simulink model "CC_CarExample_template_inclass"  (R2022b)
settings: Solver=VariableStepAuto  StartTime=0.0  StopTime=400  RelTol=1e-3
model workspace (MAT data in the model file):
  Logfile_Data_Object : robotics.ros.BagSelection (value not decoded)
  Logfile_Path = '/Users/sprinkle/Library/CloudStorage/OneDrive-Vanderbilt/Presentations/DW_JS/TEACHING/Lecture Slides/2023_09_28_16_19_25_2T3MWRFVXLW056972microstrain_record.bag'
  Logfile_Playback_Duration = nan
  Logfile_Playback_Offset = 100
libraries: robotlib x3
settings Model: Creator=sprinkle  LastModifiedBy=sprinkle
settings SolverCC: AlgebraicLoopSolver=TrustRegion
settings DebuggingCC: UnconnectedInputMsg=warning  UnconnectedOutputMsg=warning  UnconnectedLineMsg=warning  SFMachineParentedDataDiag=warning
settings ModelReferenceCC: EnableRefExpFcnMdlSchedulingChecks=on

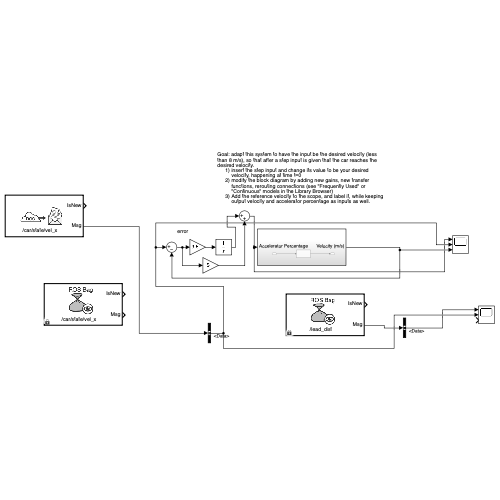
[thumbnail: Simulink's stored preview of the top-level diagram]
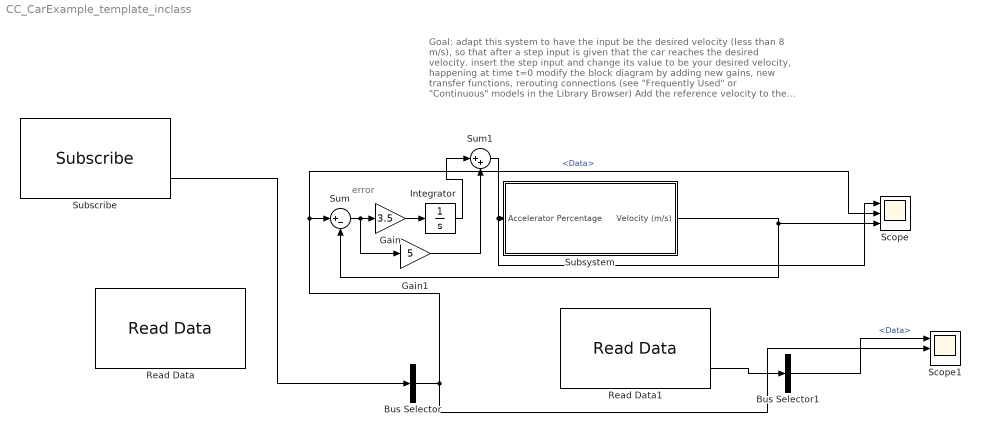
== system CC_CarExample_template_inclass ==
  note: error
  note: Goal: adapt this system to have the input be the desired velocity (less than 8 m/s), so that after a step input is given that the car reaches the desired velocity.
      | insert the step input and change its value to be your desired velocity, happening at time t=0
      | modify the block diagram by adding new gains, new transfer functions, rerouting connections (see "Frequently Used" or "Continuous" models in the Library Browser)
      | Add the reference velocity to the scope, and label it, while keeping output velocity and accelerator percentage as inputs as well.
  Bus Selector : BusSelector  OutputSignals=Data
  Bus Selector1 : BusSelector  OutputSignals=Data
  Gain : Gain  Gain=3.5
  Gain1 : Gain  Gain=5
  Integrator : Integrator
  Read Data : robotlib/Read Data  topic=/car/state/vel_x  sampleTime=-1  logfileType=rosbag  modelName=CC_CarExample_template_inclass  msgType=std_msgs/Float64
      settings: LibrarySourceBlock=roslib/ROS/Read Data
  Read Data1 : robotlib/Read Data  topic=/lead_dist  sampleTime=-1  logfileType=rosbag  modelName=CC_CarExample_template_inclass  msgType=std_msgs/Float64
      settings: LibrarySourceBlock=roslib/ROS/Read Data
  Scope : Scope  NumInputPorts=3
  Scope1 : Scope  NumInputPorts=3
  Subscribe : robotlib/Subscribe  topicSource=Specify your own  topic=/car/state/vel_x  messageType=std_msgs/Float64  SampleTime=-1  legacyBusStruct=off  messageQueueLength=1
      settings: LibrarySourceBlock=roslib/ROS/Subscribe
  Subsystem : Subsystem
  Sum : Sum  Inputs=|+-
  Sum1 : Sum  Inputs=|++
  wires:
  Subsystem/1(Velocity (m/s)) -> Scope/3, Sum/2
  Bus Selector/1 -> Scope1/2, Scope/2, Sum/1  "<Data>"
  Sum/1 -> Gain1/1, Gain/1
  Gain/1 -> Integrator/1
  Integrator/1 -> Sum1/1
  Sum1/1 -> Scope/1, Subsystem/1(Accelerator Percentage)
  Gain1/1 -> Sum1/2
  Read Data1/2 -> Bus Selector1/1
  Bus Selector1/1 -> Scope1/1  "<Data>"
  Subscribe/2 -> Bus Selector/1

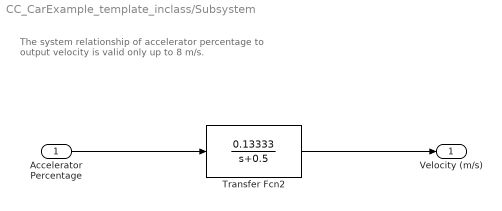
== system CC_CarExample_template_inclass/Subsystem ==
  note: The system relationship of accelerator percentage to output velocity is valid only up to 8 m/s.
  Accelerator Percentage : Inport
  Transfer Fcn2 : TransferFcn  Numerator=[0.13333]  Denominator=[1 0.5]
  Velocity (m/s) : Outport
  wires:
  Accelerator Percentage/1 -> Transfer Fcn2/1
  Transfer Fcn2/1 -> Velocity (m/s)/1
